simulink model "CCMSysServ"  (R2022b)
settings: Solver=FixedStepDiscrete  StartTime=0.0  StopTime=10.0  FixedStep=0.05  RelTol=1e-3
model workspace (MAT data in the model file):
  NvMBlockCarCfgData : Simulink.Signal (value not decoded)
  NvMBlockCarCfgDataInit : Simulink.Parameter (value not decoded)
  NvMBlockDidDataD005 : Simulink.Signal (value not decoded)
  NvMBlockDidDataD005Init : Simulink.Parameter (value not decoded)
  NvMBlockDidDataD09A : Simulink.Signal (value not decoded)
  NvMBlockDidDataD09AInit : Simulink.Parameter (value not decoded)
  NvMBlockDidDataE103 : Simulink.Signal (value not decoded)
  NvMBlockDidDataE103Init : Simulink.Parameter (value not decoded)
  NvMBlockDidDataF190 : Simulink.Signal (value not decoded)
  NvMBlockDidDataF190Init : Simulink.Parameter (value not decoded)
  NvMBlockDidDataF1AB : Simulink.Signal (value not decoded)
  NvMBlockDidDataF1ABInit : Simulink.Parameter (value not decoded)
symbols that are objects in the model workspace (value not decoded): NvMBlockCarCfgDataInit, NvMBlockDidDataD005Init, NvMBlockDidDataD09AInit, NvMBlockDidDataE103Init, NvMBlockDidDataF190Init, NvMBlockDidDataF1ABInit
(part 3 of 4)

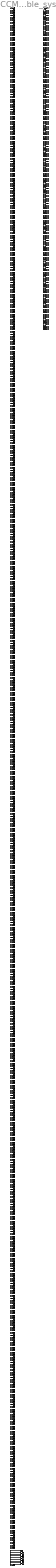
== system CCMSysServ/CCMSysServ_Runnable_sys ==
  ComMPNCState_PNCState_read : Inport
  ComMPNCState_VFCState_read : Inport  Port=2
  EcuKeepActv_EcuKeepActv_read : Inport  Port=3
  CarTiGlb_CarTiGlb_read : Inport  Port=4
  CarTiGlb_CarTiGlb_IsUpdated_read : Inport  Port=5
  ActrFrntReqForRowFirstLeModDistbn_ActrFrntReqForRowFirstLeModDistbn_read : Inport  Port=6
  CarCfg_CarCfg1_read : Inport  Port=7
  CarCfg_CarCfg10_read : Inport  Port=8
  CarCfg_CarCfg100_read : Inport  Port=9
  CarCfg_CarCfg1002_read : Inport  Port=10
  CarCfg_CarCfg1004_read : Inport  Port=11
  CarCfg_CarCfg1007_read : Inport  Port=12
  CarCfg_CarCfg101_read : Inport  Port=13
  CarCfg_CarCfg1011_read : Inport  Port=14
  CarCfg_CarCfg1017_read : Inport  Port=15
  CarCfg_CarCfg1018_read : Inport  Port=16
  CarCfg_CarCfg102_read : Inport  Port=17
  CarCfg_CarCfg103_read : Inport  Port=18
  CarCfg_CarCfg1034_read : Inport  Port=19
  CarCfg_CarCfg1038_read : Inport  Port=20
  CarCfg_CarCfg1039_read : Inport  Port=21
  CarCfg_CarCfg104_read : Inport  Port=22
  CarCfg_CarCfg1042_read : Inport  Port=23
  CarCfg_CarCfg1043_read : Inport  Port=24
  CarCfg_CarCfg1044_read : Inport  Port=25
  CarCfg_CarCfg1045_read : Inport  Port=26
  CarCfg_CarCfg1047_read : Inport  Port=27
  CarCfg_CarCfg105_read : Inport  Port=28
  CarCfg_CarCfg1052_read : Inport  Port=29
  CarCfg_CarCfg1057_read : Inport  Port=30
  CarCfg_CarCfg106_read : Inport  Port=31
  CarCfg_CarCfg1061_read : Inport  Port=32
  CarCfg_CarCfg1063_read : Inport  Port=33
  CarCfg_CarCfg1065_read : Inport  Port=34
  CarCfg_CarCfg107_read : Inport  Port=35
  CarCfg_CarCfg1075_read : Inport  Port=36
  CarCfg_CarCfg1077_read : Inport  Port=37
  CarCfg_CarCfg108_read : Inport  Port=38
  CarCfg_CarCfg1080_read : Inport  Port=39
  CarCfg_CarCfg1083_read : Inport  Port=40
  CarCfg_CarCfg1087_read : Inport  Port=41
  CarCfg_CarCfg1088_read : Inport  Port=42
  CarCfg_CarCfg109_read : Inport  Port=43
  CarCfg_CarCfg1090_read : Inport  Port=44
  CarCfg_CarCfg1096_read : Inport  Port=45
  CarCfg_CarCfg110_read : Inport  Port=46
  CarCfg_CarCfg1101_read : Inport  Port=47
  CarCfg_CarCfg1102_read : Inport  Port=48
  CarCfg_CarCfg1103_read : Inport  Port=49
  CarCfg_CarCfg1104_read : Inport  Port=50
  CarCfg_CarCfg111_read : Inport  Port=51
  CarCfg_CarCfg1111_read : Inport  Port=52
  CarCfg_CarCfg1112_read : Inport  Port=53
  CarCfg_CarCfg112_read : Inport  Port=54
  CarCfg_CarCfg113_read : Inport  Port=55
  CarCfg_CarCfg1130_read : Inport  Port=56
  CarCfg_CarCfg1134_read : Inport  Port=57
  CarCfg_CarCfg1136_read : Inport  Port=58
  CarCfg_CarCfg114_read : Inport  Port=59
  CarCfg_CarCfg115_read : Inport  Port=60
  CarCfg_CarCfg1154_read : Inport  Port=61
  CarCfg_CarCfg116_read : Inport  Port=62
  CarCfg_CarCfg1166_read : Inport  Port=63
  CarCfg_CarCfg117_read : Inport  Port=64
  CarCfg_CarCfg1170_read : Inport  Port=65
  CarCfg_CarCfg1171_read : Inport  Port=66
  CarCfg_CarCfg1172_read : Inport  Port=67
  CarCfg_CarCfg1173_read : Inport  Port=68
  CarCfg_CarCfg1174_read : Inport  Port=69
  CarCfg_CarCfg1175_read : Inport  Port=70
  CarCfg_CarCfg1177_read : Inport  Port=71
  CarCfg_CarCfg1178_read : Inport  Port=72
  CarCfg_CarCfg118_read : Inport  Port=73
  CarCfg_CarCfg1184_read : Inport  Port=74
  CarCfg_CarCfg1185_read : Inport  Port=75
  CarCfg_CarCfg1189_read : Inport  Port=76
  CarCfg_CarCfg119_read : Inport  Port=77
  CarCfg_CarCfg12_read : Inport  Port=78
  CarCfg_CarCfg120_read : Inport  Port=79
  CarCfg_CarCfg121_read : Inport  Port=80
  CarCfg_CarCfg1214_read : Inport  Port=81
  CarCfg_CarCfg1216_read : Inport  Port=82
  CarCfg_CarCfg1217_read : Inport  Port=83
  CarCfg_CarCfg1218_read : Inport  Port=84
  CarCfg_CarCfg1219_read : Inport  Port=85
  CarCfg_CarCfg122_read : Inport  Port=86
  CarCfg_CarCfg123_read : Inport  Port=87
  CarCfg_CarCfg1230_read : Inport  Port=88
  CarCfg_CarCfg1231_read : Inport  Port=89
  CarCfg_CarCfg1232_read : Inport  Port=90
  CarCfg_CarCfg1233_read : Inport  Port=91
  CarCfg_CarCfg1234_read : Inport  Port=92
  CarCfg_CarCfg124_read : Inport  Port=93
  CarCfg_CarCfg1247_read : Inport  Port=94
  CarCfg_CarCfg125_read : Inport  Port=95
  CarCfg_CarCfg1250_read : Inport  Port=96
  CarCfg_CarCfg1251_read : Inport  Port=97
  CarCfg_CarCfg1258_read : Inport  Port=98
  CarCfg_CarCfg1259_read : Inport  Port=99
  CarCfg_CarCfg126_read : Inport  Port=100
  CarCfg_CarCfg1260_read : Inport  Port=101
  CarCfg_CarCfg1261_read : Inport  Port=102
  CarCfg_CarCfg1264_read : Inport  Port=103
  CarCfg_CarCfg1266_read : Inport  Port=104
  CarCfg_CarCfg1267_read : Inport  Port=105
  CarCfg_CarCfg127_read : Inport  Port=106
  CarCfg_CarCfg1273_read : Inport  Port=107
  CarCfg_CarCfg1275_read : Inport  Port=108
  CarCfg_CarCfg1277_read : Inport  Port=109
  CarCfg_CarCfg1278_read : Inport  Port=110
  CarCfg_CarCfg1279_read : Inport  Port=111
  CarCfg_CarCfg128_read : Inport  Port=112
  CarCfg_CarCfg1283_read : Inport  Port=113
  CarCfg_CarCfg1285_read : Inport  Port=114
  CarCfg_CarCfg1286_read : Inport  Port=115
  CarCfg_CarCfg1287_read : Inport  Port=116
  CarCfg_CarCfg129_read : Inport  Port=117
  CarCfg_CarCfg13_read : Inport  Port=118
  CarCfg_CarCfg130_read : Inport  Port=119
  CarCfg_CarCfg131_read : Inport  Port=120
  CarCfg_CarCfg132_read : Inport  Port=121
  CarCfg_CarCfg133_read : Inport  Port=122
  CarCfg_CarCfg134_read : Inport  Port=123
  CarCfg_CarCfg135_read : Inport  Port=124
  CarCfg_CarCfg136_read : Inport  Port=125
  CarCfg_CarCfg137_read : Inport  Port=126
  CarCfg_CarCfg138_read : Inport  Port=127
  CarCfg_CarCfg139_read : Inport  Port=128
  CarCfg_CarCfg14_read : Inport  Port=129
  CarCfg_CarCfg140_read : Inport  Port=130
  CarCfg_CarCfg141_read : Inport  Port=131
  CarCfg_CarCfg142_read : Inport  Port=132
  CarCfg_CarCfg143_read : Inport  Port=133
  CarCfg_CarCfg144_read : Inport  Port=134
  CarCfg_CarCfg145_read : Inport  Port=135
  CarCfg_CarCfg146_read : Inport  Port=136
  CarCfg_CarCfg148_read : Inport  Port=137
  CarCfg_CarCfg149_read : Inport  Port=138
  CarCfg_CarCfg150_read : Inport  Port=139
  CarCfg_CarCfg151_read : Inport  Port=140
  CarCfg_CarCfg152_read : Inport  Port=141
  CarCfg_CarCfg153_read : Inport  Port=142
  CarCfg_CarCfg154_read : Inport  Port=143
  CarCfg_CarCfg155_read : Inport  Port=144
  CarCfg_CarCfg156_read : Inport  Port=145
  CarCfg_CarCfg157_read : Inport  Port=146
  CarCfg_CarCfg158_read : Inport  Port=147
  CarCfg_CarCfg159_read : Inport  Port=148
  CarCfg_CarCfg16_read : Inport  Port=149
  CarCfg_CarCfg160_read : Inport  Port=150
  CarCfg_CarCfg161_read : Inport  Port=151
  CarCfg_CarCfg162_read : Inport  Port=152
  CarCfg_CarCfg163_read : Inport  Port=153
  CarCfg_CarCfg164_read : Inport  Port=154
  CarCfg_CarCfg165_read : Inport  Port=155
  CarCfg_CarCfg166_read : Inport  Port=156
  CarCfg_CarCfg167_read : Inport  Port=157
  CarCfg_CarCfg168_read : Inport  Port=158
  CarCfg_CarCfg169_read : Inport  Port=159
  CarCfg_CarCfg17_read : Inport  Port=160
  CarCfg_CarCfg170_read : Inport  Port=161
  CarCfg_CarCfg171_read : Inport  Port=162
  CarCfg_CarCfg172_read : Inport  Port=163
  CarCfg_CarCfg173_read : Inport  Port=164
  CarCfg_CarCfg174_read : Inport  Port=165
  CarCfg_CarCfg175_read : Inport  Port=166
  CarCfg_CarCfg176_read : Inport  Port=167
  CarCfg_CarCfg177_read : Inport  Port=168
  CarCfg_CarCfg178_read : Inport  Port=169
  CarCfg_CarCfg179_read : Inport  Port=170
  CarCfg_CarCfg18_read : Inport  Port=171
  CarCfg_CarCfg180_read : Inport  Port=172
  CarCfg_CarCfg181_read : Inport  Port=173
  CarCfg_CarCfg182_read : Inport  Port=174
  CarCfg_CarCfg183_read : Inport  Port=175
  CarCfg_CarCfg184_read : Inport  Port=176
  CarCfg_CarCfg185_read : Inport  Port=177
  CarCfg_CarCfg186_read : Inport  Port=178
  CarCfg_CarCfg187_read : Inport  Port=179
  CarCfg_CarCfg188_read : Inport  Port=180
  CarCfg_CarCfg189_read : Inport  Port=181
  CarCfg_CarCfg19_read : Inport  Port=182
  CarCfg_CarCfg190_read : Inport  Port=183
  CarCfg_CarCfg191_read : Inport  Port=184
  CarCfg_CarCfg192_read : Inport  Port=185
  CarCfg_CarCfg193_read : Inport  Port=186
  CarCfg_CarCfg194_read : Inport  Port=187
  CarCfg_CarCfg195_read : Inport  Port=188
  CarCfg_CarCfg196_read : Inport  Port=189
  CarCfg_CarCfg197_read : Inport  Port=190
  CarCfg_CarCfg198_read : Inport  Port=191
  CarCfg_CarCfg199_read : Inport  Port=192
  CarCfg_CarCfg2_read : Inport  Port=193
  CarCfg_CarCfg20_read : Inport  Port=194
  CarCfg_CarCfg200_read : Inport  Port=195
  CarCfg_CarCfg201_read : Inport  Port=196
  CarCfg_CarCfg202_read : Inport  Port=197
  CarCfg_CarCfg203_read : Inport  Port=198
  CarCfg_CarCfg204_read : Inport  Port=199
  CarCfg_CarCfg205_read : Inport  Port=200
  CarCfg_CarCfg206_read : Inport  Port=201
  CarCfg_CarCfg207_read : Inport  Port=202
  CarCfg_CarCfg208_read : Inport  Port=203
  CarCfg_CarCfg209_read : Inport  Port=204
  CarCfg_CarCfg210_read : Inport  Port=205
  CarCfg_CarCfg211_read : Inport  Port=206
  CarCfg_CarCfg212_read : Inport  Port=207
  CarCfg_CarCfg213_read : Inport  Port=208
  CarCfg_CarCfg214_read : Inport  Port=209
  CarCfg_CarCfg215_read : Inport  Port=210
  CarCfg_CarCfg216_read : Inport  Port=211
  CarCfg_CarCfg217_read : Inport  Port=212
  CarCfg_CarCfg218_read : Inport  Port=213
  CarCfg_CarCfg219_read : Inport  Port=214
  CarCfg_CarCfg22_read : Inport  Port=215
  CarCfg_CarCfg220_read : Inport  Port=216
  CarCfg_CarCfg221_read : Inport  Port=217
  CarCfg_CarCfg222_read : Inport  Port=218
  CarCfg_CarCfg223_read : Inport  Port=219
  CarCfg_CarCfg224_read : Inport  Port=220
  CarCfg_CarCfg225_read : Inport  Port=221
  CarCfg_CarCfg226_read : Inport  Port=222
  CarCfg_CarCfg227_read : Inport  Port=223
  CarCfg_CarCfg228_read : Inport  Port=224
  CarCfg_CarCfg229_read : Inport  Port=225
  CarCfg_CarCfg23_read : Inport  Port=226
  CarCfg_CarCfg230_read : Inport  Port=227
  CarCfg_CarCfg231_read : Inport  Port=228
  CarCfg_CarCfg232_read : Inport  Port=229
  CarCfg_CarCfg235_read : Inport  Port=230
  CarCfg_CarCfg236_read : Inport  Port=231
  CarCfg_CarCfg237_read : Inport  Port=232
  CarCfg_CarCfg238_read : Inport  Port=233
  CarCfg_CarCfg239_read : Inport  Port=234
  CarCfg_CarCfg24_read : Inport  Port=235
  CarCfg_CarCfg240_read : Inport  Port=236
  CarCfg_CarCfg241_read : Inport  Port=237
  CarCfg_CarCfg242_read : Inport  Port=238
  CarCfg_CarCfg243_read : Inport  Port=239
  CarCfg_CarCfg244_read : Inport  Port=240
  CarCfg_CarCfg245_read : Inport  Port=241
  CarCfg_CarCfg246_read : Inport  Port=242
  CarCfg_CarCfg247_read : Inport  Port=243
  CarCfg_CarCfg248_read : Inport  Port=244
  CarCfg_CarCfg249_read : Inport  Port=245
  CarCfg_CarCfg25_read : Inport  Port=246
  CarCfg_CarCfg250_read : Inport  Port=247
  CarCfg_CarCfg253_read : Inport  Port=248
  CarCfg_CarCfg254_read : Inport  Port=249
  CarCfg_CarCfg255_read : Inport  Port=250
  CarCfg_CarCfg256_read : Inport  Port=251
  CarCfg_CarCfg257_read : Inport  Port=252
  CarCfg_CarCfg258_read : Inport  Port=253
  CarCfg_CarCfg259_read : Inport  Port=254
  CarCfg_CarCfg262_read : Inport  Port=255
  CarCfg_CarCfg263_read : Inport  Port=256
  CarCfg_CarCfg267_read : Inport  Port=257
  CarCfg_CarCfg268_read : Inport  Port=258
  CarCfg_CarCfg270_read : Inport  Port=259
  CarCfg_CarCfg271_read : Inport  Port=260
  CarCfg_CarCfg273_read : Inport  Port=261
  CarCfg_CarCfg274_read : Inport  Port=262
  CarCfg_CarCfg275_read : Inport  Port=263
  CarCfg_CarCfg28_read : Inport  Port=264
  CarCfg_CarCfg289_read : Inport  Port=265
  CarCfg_CarCfg290_read : Inport  Port=266
  CarCfg_CarCfg3_read : Inport  Port=267
  CarCfg_CarCfg30_read : Inport  Port=268
  CarCfg_CarCfg301_read : Inport  Port=269
  CarCfg_CarCfg302_read : Inport  Port=270
  CarCfg_CarCfg303_read : Inport  Port=271
  CarCfg_CarCfg304_read : Inport  Port=272
  CarCfg_CarCfg305_read : Inport  Port=273
  CarCfg_CarCfg307_read : Inport  Port=274
  CarCfg_CarCfg308_read : Inport  Port=275
  CarCfg_CarCfg309_read : Inport  Port=276
  CarCfg_CarCfg310_read : Inport  Port=277
  CarCfg_CarCfg311_read : Inport  Port=278
  CarCfg_CarCfg312_read : Inport  Port=279
  CarCfg_CarCfg313_read : Inport  Port=280
  CarCfg_CarCfg314_read : Inport  Port=281
  CarCfg_CarCfg315_read : Inport  Port=282
  CarCfg_CarCfg316_read : Inport  Port=283
  CarCfg_CarCfg317_read : Inport  Port=284
  CarCfg_CarCfg318_read : Inport  Port=285
  CarCfg_CarCfg319_read : Inport  Port=286
  CarCfg_CarCfg32_read : Inport  Port=287
  CarCfg_CarCfg321_read : Inport  Port=288
  CarCfg_CarCfg322_read : Inport  Port=289
  CarCfg_CarCfg323_read : Inport  Port=290
  CarCfg_CarCfg324_read : Inport  Port=291
  CarCfg_CarCfg325_read : Inport  Port=292
  CarCfg_CarCfg33_read : Inport  Port=293
  CarCfg_CarCfg335_read : Inport  Port=294
  CarCfg_CarCfg336_read : Inport  Port=295
  CarCfg_CarCfg337_read : Inport  Port=296
  CarCfg_CarCfg338_read : Inport  Port=297
  CarCfg_CarCfg339_read : Inport  Port=298
  CarCfg_CarCfg34_read : Inport  Port=299
  CarCfg_CarCfg340_read : Inport  Port=300
  CarCfg_CarCfg341_read : Inport  Port=301
  CarCfg_CarCfg342_read : Inport  Port=302
  CarCfg_CarCfg343_read : Inport  Port=303
  CarCfg_CarCfg344_read : Inport  Port=304
  CarCfg_CarCfg345_read : Inport  Port=305
  CarCfg_CarCfg346_read : Inport  Port=306
  CarCfg_CarCfg347_read : Inport  Port=307
  CarCfg_CarCfg348_read : Inport  Port=308
  CarCfg_CarCfg349_read : Inport  Port=309
  CarCfg_CarCfg35_read : Inport  Port=310
  CarCfg_CarCfg350_read : Inport  Port=311
  CarCfg_CarCfg351_read : Inport  Port=312
  CarCfg_CarCfg352_read : Inport  Port=313
  CarCfg_CarCfg353_read : Inport  Port=314
  CarCfg_CarCfg354_read : Inport  Port=315
  CarCfg_CarCfg355_read : Inport  Port=316
  CarCfg_CarCfg356_read : Inport  Port=317
  CarCfg_CarCfg357_read : Inport  Port=318
  CarCfg_CarCfg358_read : Inport  Port=319
  CarCfg_CarCfg359_read : Inport  Port=320
  CarCfg_CarCfg36_read : Inport  Port=321
  CarCfg_CarCfg360_read : Inport  Port=322
  CarCfg_CarCfg361_read : Inport  Port=323
  CarCfg_CarCfg362_read : Inport  Port=324
  CarCfg_CarCfg363_read : Inport  Port=325
  CarCfg_CarCfg364_read : Inport  Port=326
  CarCfg_CarCfg365_read : Inport  Port=327
  CarCfg_CarCfg366_read : Inport  Port=328
  CarCfg_CarCfg367_read : Inport  Port=329
  CarCfg_CarCfg368_read : Inport  Port=330
  CarCfg_CarCfg369_read : Inport  Port=331
  CarCfg_CarCfg37_read : Inport  Port=332
  CarCfg_CarCfg371_read : Inport  Port=333
  CarCfg_CarCfg372_read : Inport  Port=334
  CarCfg_CarCfg373_read : Inport  Port=335
  CarCfg_CarCfg374_read : Inport  Port=336
  CarCfg_CarCfg376_read : Inport  Port=337
  CarCfg_CarCfg38_read : Inport  Port=338
  CarCfg_CarCfg39_read : Inport  Port=339
  CarCfg_CarCfg4_read : Inport  Port=340
  CarCfg_CarCfg401_read : Inport  Port=341
  CarCfg_CarCfg407_read : Inport  Port=342
  CarCfg_CarCfg408_read : Inport  Port=343
  CarCfg_CarCfg409_read : Inport  Port=344
  CarCfg_CarCfg41_read : Inport  Port=345
  CarCfg_CarCfg410_read : Inport  Port=346
  CarCfg_CarCfg413_read : Inport  Port=347
  CarCfg_CarCfg414_read : Inport  Port=348
  CarCfg_CarCfg415_read : Inport  Port=349
  CarCfg_CarCfg42_read : Inport  Port=350
  CarCfg_CarCfg43_read : Inport  Port=351
  CarCfg_CarCfg44_read : Inport  Port=352
  CarCfg_CarCfg441_read : Inport  Port=353
  CarCfg_CarCfg449_read : Inport  Port=354
  CarCfg_CarCfg45_read : Inport  Port=355
  CarCfg_CarCfg450_read : Inport  Port=356
  CarCfg_CarCfg451_read : Inport  Port=357
  CarCfg_CarCfg452_read : Inport  Port=358
  CarCfg_CarCfg453_read : Inport  Port=359
  CarCfg_CarCfg454_read : Inport  Port=360
  CarCfg_CarCfg455_read : Inport  Port=361
  CarCfg_CarCfg456_read : Inport  Port=362
  CarCfg_CarCfg457_read : Inport  Port=363
  CarCfg_CarCfg458_read : Inport  Port=364
  CarCfg_CarCfg459_read : Inport  Port=365
  CarCfg_CarCfg46_read : Inport  Port=366
  CarCfg_CarCfg460_read : Inport  Port=367
  CarCfg_CarCfg461_read : Inport  Port=368
  CarCfg_CarCfg462_read : Inport  Port=369
  CarCfg_CarCfg463_read : Inport  Port=370
  CarCfg_CarCfg464_read : Inport  Port=371
  CarCfg_CarCfg465_read : Inport  Port=372
  CarCfg_CarCfg466_read : Inport  Port=373
  CarCfg_CarCfg467_read : Inport  Port=374
  CarCfg_CarCfg468_read : Inport  Port=375
  CarCfg_CarCfg469_read : Inport  Port=376
  CarCfg_CarCfg47_read : Inport  Port=377
  CarCfg_CarCfg470_read : Inport  Port=378
  CarCfg_CarCfg471_read : Inport  Port=379
  CarCfg_CarCfg472_read : Inport  Port=380
  CarCfg_CarCfg473_read : Inport  Port=381
  CarCfg_CarCfg474_read : Inport  Port=382
  CarCfg_CarCfg475_read : Inport  Port=383
  CarCfg_CarCfg476_read : Inport  Port=384
  CarCfg_CarCfg477_read : Inport  Port=385
  CarCfg_CarCfg478_read : Inport  Port=386
  CarCfg_CarCfg479_read : Inport  Port=387
  CarCfg_CarCfg48_read : Inport  Port=388
  CarCfg_CarCfg480_read : Inport  Port=389
  CarCfg_CarCfg481_read : Inport  Port=390
  CarCfg_CarCfg482_read : Inport  Port=391
  CarCfg_CarCfg483_read : Inport  Port=392
  CarCfg_CarCfg484_read : Inport  Port=393
  CarCfg_CarCfg485_read : Inport  Port=394
  CarCfg_CarCfg486_read : Inport  Port=395
  CarCfg_CarCfg487_read : Inport  Port=396
  CarCfg_CarCfg488_read : Inport  Port=397
  CarCfg_CarCfg489_read : Inport  Port=398
  CarCfg_CarCfg49_read : Inport  Port=399
  CarCfg_CarCfg490_read : Inport  Port=400
  CarCfg_CarCfg491_read : Inport  Port=401
  CarCfg_CarCfg493_read : Inport  Port=402
  CarCfg_CarCfg494_read : Inport  Port=403
  CarCfg_CarCfg495_read : Inport  Port=404
  CarCfg_CarCfg496_read : Inport  Port=405
  CarCfg_CarCfg497_read : Inport  Port=406
  CarCfg_CarCfg498_read : Inport  Port=407
  CarCfg_CarCfg499_read : Inport  Port=408
  CarCfg_CarCfg5_read : Inport  Port=409
  CarCfg_CarCfg50_read : Inport  Port=410
  CarCfg_CarCfg500_read : Inport  Port=411
  CarCfg_CarCfg501_read : Inport  Port=412
  CarCfg_CarCfg502_read : Inport  Port=413
  CarCfg_CarCfg503_read : Inport  Port=414
  CarCfg_CarCfg504_read : Inport  Port=415
  CarCfg_CarCfg505_read : Inport  Port=416
  CarCfg_CarCfg506_read : Inport  Port=417
  CarCfg_CarCfg507_read : Inport  Port=418
  CarCfg_CarCfg508_read : Inport  Port=419
  CarCfg_CarCfg509_read : Inport  Port=420
  CarCfg_CarCfg51_read : Inport  Port=421
  CarCfg_CarCfg510_read : Inport  Port=422
  CarCfg_CarCfg511_read : Inport  Port=423
  CarCfg_CarCfg512_read : Inport  Port=424
  CarCfg_CarCfg513_read : Inport  Port=425
  CarCfg_CarCfg514_read : Inport  Port=426
  CarCfg_CarCfg515_read : Inport  Port=427
  CarCfg_CarCfg516_read : Inport  Port=428
  CarCfg_CarCfg517_read : Inport  Port=429
  CarCfg_CarCfg518_read : Inport  Port=430
  CarCfg_CarCfg519_read : Inport  Port=431
  CarCfg_CarCfg52_read : Inport  Port=432
  CarCfg_CarCfg520_read : Inport  Port=433
  CarCfg_CarCfg521_read : Inport  Port=434
  CarCfg_CarCfg522_read : Inport  Port=435
  CarCfg_CarCfg523_read : Inport  Port=436
  CarCfg_CarCfg524_read : Inport  Port=437
  CarCfg_CarCfg525_read : Inport  Port=438
  CarCfg_CarCfg526_read : Inport  Port=439
  CarCfg_CarCfg527_read : Inport  Port=440
  CarCfg_CarCfg528_read : Inport  Port=441
  CarCfg_CarCfg529_read : Inport  Port=442
  CarCfg_CarCfg53_read : Inport  Port=443
  CarCfg_CarCfg531_read : Inport  Port=444
  CarCfg_CarCfg532_read : Inport  Port=445
  CarCfg_CarCfg533_read : Inport  Port=446
  CarCfg_CarCfg534_read : Inport  Port=447
  CarCfg_CarCfg535_read : Inport  Port=448
  CarCfg_CarCfg536_read : Inport  Port=449
  CarCfg_CarCfg54_read : Inport  Port=450
  CarCfg_CarCfg540_read : Inport  Port=451
  CarCfg_CarCfg541_read : Inport  Port=452
  CarCfg_CarCfg543_read : Inport  Port=453
  CarCfg_CarCfg544_read : Inport  Port=454
  CarCfg_CarCfg545_read : Inport  Port=455
  CarCfg_CarCfg546_read : Inport  Port=456
  CarCfg_CarCfg547_read : Inport  Port=457
  CarCfg_CarCfg55_read : Inport  Port=458
  CarCfg_CarCfg550_read : Inport  Port=459
  CarCfg_CarCfg551_read : Inport  Port=460
  CarCfg_CarCfg56_read : Inport  Port=461
  CarCfg_CarCfg561_read : Inport  Port=462
  CarCfg_CarCfg564_read : Inport  Port=463
  CarCfg_CarCfg565_read : Inport  Port=464
  CarCfg_CarCfg566_read : Inport  Port=465
  CarCfg_CarCfg567_read : Inport  Port=466
  CarCfg_CarCfg568_read : Inport  Port=467
  CarCfg_CarCfg569_read : Inport  Port=468
  CarCfg_CarCfg57_read : Inport  Port=469
  CarCfg_CarCfg578_read : Inport  Port=470
  CarCfg_CarCfg579_read : Inport  Port=471
  CarCfg_CarCfg58_read : Inport  Port=472
  CarCfg_CarCfg580_read : Inport  Port=473
  CarCfg_CarCfg59_read : Inport  Port=474
  CarCfg_CarCfg590_read : Inport  Port=475
  CarCfg_CarCfg591_read : Inport  Port=476
  CarCfg_CarCfg593_read : Inport  Port=477
  CarCfg_CarCfg595_read : Inport  Port=478
  CarCfg_CarCfg599_read : Inport  Port=479
  CarCfg_CarCfg6_read : Inport  Port=480
  CarCfg_CarCfg60_read : Inport  Port=481
  CarCfg_CarCfg604_read : Inport  Port=482
  CarCfg_CarCfg609_read : Inport  Port=483
  CarCfg_CarCfg61_read : Inport  Port=484
  CarCfg_CarCfg611_read : Inport  Port=485
  CarCfg_CarCfg613_read : Inport  Port=486
  CarCfg_CarCfg615_read : Inport  Port=487
  CarCfg_CarCfg616_read : Inport  Port=488
  CarCfg_CarCfg617_read : Inport  Port=489
  CarCfg_CarCfg618_read : Inport  Port=490
  CarCfg_CarCfg62_read : Inport  Port=491
  CarCfg_CarCfg622_read : Inport  Port=492
  CarCfg_CarCfg624_read : Inport  Port=493
  CarCfg_CarCfg626_read : Inport  Port=494
  CarCfg_CarCfg627_read : Inport  Port=495
  CarCfg_CarCfg628_read : Inport  Port=496
  CarCfg_CarCfg629_read : Inport  Port=497
  CarCfg_CarCfg63_read : Inport  Port=498
  CarCfg_CarCfg630_read : Inport  Port=499
  CarCfg_CarCfg634_read : Inport  Port=500
  CarCfg_CarCfg635_read : Inport  Port=501
  CarCfg_CarCfg639_read : Inport  Port=502
  CarCfg_CarCfg64_read : Inport  Port=503
  CarCfg_CarCfg640_read : Inport  Port=504
  CarCfg_CarCfg642_read : Inport  Port=505
  CarCfg_CarCfg644_read : Inport  Port=506
  CarCfg_CarCfg645_read : Inport  Port=507
  CarCfg_CarCfg646_read : Inport  Port=508
  CarCfg_CarCfg647_read : Inport  Port=509
  CarCfg_CarCfg648_read : Inport  Port=510
  CarCfg_CarCfg65_read : Inport  Port=511
  CarCfg_CarCfg650_read : Inport  Port=512
  CarCfg_CarCfg651_read : Inport  Port=513
  CarCfg_CarCfg652_read : Inport  Port=514
  CarCfg_CarCfg659_read : Inport  Port=515
  CarCfg_CarCfg66_read : Inport  Port=516
  CarCfg_CarCfg660_read : Inport  Port=517
  CarCfg_CarCfg665_read : Inport  Port=518
  CarCfg_CarCfg67_read : Inport  Port=519
  CarCfg_CarCfg671_read : Inport  Port=520
  CarCfg_CarCfg672_read : Inport  Port=521
  CarCfg_CarCfg675_read : Inport  Port=522
  CarCfg_CarCfg677_read : Inport  Port=523
  CarCfg_CarCfg678_read : Inport  Port=524
  CarCfg_CarCfg68_read : Inport  Port=525
  CarCfg_CarCfg683_read : Inport  Port=526
  CarCfg_CarCfg684_read : Inport  Port=527
  CarCfg_CarCfg686_read : Inport  Port=528
  CarCfg_CarCfg687_read : Inport  Port=529
  CarCfg_CarCfg688_read : Inport  Port=530
  CarCfg_CarCfg69_read : Inport  Port=531
  CarCfg_CarCfg695_read : Inport  Port=532
  CarCfg_CarCfg697_read : Inport  Port=533
  CarCfg_CarCfg698_read : Inport  Port=534
  CarCfg_CarCfg699_read : Inport  Port=535
  CarCfg_CarCfg70_read : Inport  Port=536
  CarCfg_CarCfg701_read : Inport  Port=537
  CarCfg_CarCfg705_read : Inport  Port=538
  CarCfg_CarCfg709_read : Inport  Port=539
  CarCfg_CarCfg71_read : Inport  Port=540
  CarCfg_CarCfg714_read : Inport  Port=541
  CarCfg_CarCfg715_read : Inport  Port=542
  CarCfg_CarCfg718_read : Inport  Port=543
  CarCfg_CarCfg719_read : Inport  Port=544
  CarCfg_CarCfg72_read : Inport  Port=545
  CarCfg_CarCfg720_read : Inport  Port=546
  CarCfg_CarCfg721_read : Inport  Port=547
  CarCfg_CarCfg726_read : Inport  Port=548
  CarCfg_CarCfg73_read : Inport  Port=549
  CarCfg_CarCfg732_read : Inport  Port=550
  CarCfg_CarCfg733_read : Inport  Port=551
  CarCfg_CarCfg734_read : Inport  Port=552
  CarCfg_CarCfg74_read : Inport  Port=553
  CarCfg_CarCfg740_read : Inport  Port=554
  CarCfg_CarCfg741_read : Inport  Port=555
  CarCfg_CarCfg742_read : Inport  Port=556
  CarCfg_CarCfg746_read : Inport  Port=557
  CarCfg_CarCfg75_read : Inport  Port=558
  CarCfg_CarCfg753_read : Inport  Port=559
  CarCfg_CarCfg755_read : Inport  Port=560
  CarCfg_CarCfg756_read : Inport  Port=561
  CarCfg_CarCfg76_read : Inport  Port=562
  CarCfg_CarCfg763_read : Inport  Port=563
  CarCfg_CarCfg766_read : Inport  Port=564
  CarCfg_CarCfg768_read : Inport  Port=565
  CarCfg_CarCfg77_read : Inport  Port=566
  CarCfg_CarCfg770_read : Inport  Port=567
  CarCfg_CarCfg773_read : Inport  Port=568
  CarCfg_CarCfg776_read : Inport  Port=569
  CarCfg_CarCfg779_read : Inport  Port=570
  CarCfg_CarCfg78_read : Inport  Port=571
  CarCfg_CarCfg787_read : Inport  Port=572
  CarCfg_CarCfg789_read : Inport  Port=573
  CarCfg_CarCfg79_read : Inport  Port=574
  CarCfg_CarCfg790_read : Inport  Port=575
  CarCfg_CarCfg793_read : Inport  Port=576
  CarCfg_CarCfg794_read : Inport  Port=577
  CarCfg_CarCfg798_read : Inport  Port=578
  CarCfg_CarCfg8_read : Inport  Port=579
  CarCfg_CarCfg80_read : Inport  Port=580
  CarCfg_CarCfg81_read : Inport  Port=581
  CarCfg_CarCfg811_read : Inport  Port=582
  CarCfg_CarCfg814_read : Inport  Port=583
  CarCfg_CarCfg816_read : Inport  Port=584
  CarCfg_CarCfg82_read : Inport  Port=585
  CarCfg_CarCfg822_read : Inport  Port=586
  CarCfg_CarCfg826_read : Inport  Port=587
  CarCfg_CarCfg829_read : Inport  Port=588
  CarCfg_CarCfg83_read : Inport  Port=589
  CarCfg_CarCfg831_read : Inport  Port=590
  CarCfg_CarCfg834_read : Inport  Port=591
  CarCfg_CarCfg835_read : Inport  Port=592
  CarCfg_CarCfg837_read : Inport  Port=593
  CarCfg_CarCfg839_read : Inport  Port=594
  CarCfg_CarCfg84_read : Inport  Port=595
  CarCfg_CarCfg841_read : Inport  Port=596
  CarCfg_CarCfg842_read : Inport  Port=597
  CarCfg_CarCfg846_read : Inport  Port=598
  CarCfg_CarCfg848_read : Inport  Port=599
  CarCfg_CarCfg849_read : Inport  Port=600
  CarCfg_CarCfg85_read : Inport  Port=601
  CarCfg_CarCfg851_read : Inport  Port=602
  CarCfg_CarCfg855_read : Inport  Port=603
  CarCfg_CarCfg857_read : Inport  Port=604
  CarCfg_CarCfg858_read : Inport  Port=605
  CarCfg_CarCfg86_read : Inport  Port=606
  CarCfg_CarCfg87_read : Inport  Port=607
  CarCfg_CarCfg88_read : Inport  Port=608
  CarCfg_CarCfg89_read : Inport  Port=609
  CarCfg_CarCfg9_read : Inport  Port=610
  CarCfg_CarCfg90_read : Inport  Port=611
  CarCfg_CarCfg91_read : Inport  Port=612
  CarCfg_CarCfg92_read : Inport  Port=613
  CarCfg_CarCfg93_read : Inport  Port=614
  CarCfg_CarCfg94_read : Inport  Port=615
  CarCfg_CarCfg95_read : Inport  Port=616
  CarCfg_CarCfg96_read : Inport  Port=617
  CarCfg_CarCfg97_read : Inport  Port=618
  CarCfg_CarCfg98_read : Inport  Port=619
  CarCfg_CarCfg99_read : Inport  Port=620
  VehCfgPrm_VehCfgPrm_read : Inport  Port=621
  VehCfgPrmExt_VehCfgPrmExt_read : Inport  Port=622
  VehModMngtGlbSafe1_VehModMngtGlbSafe1_read : Inport  Port=623
  Vin_Vin_read : Inport  Port=624
  BswLifeCycle_LifeCycle_read : Inport  Port=625
  function : TriggerPort  TriggerType=function-call  VariantControl=(inherit)  SampleTimeType=periodic  SampleTime=-1
  Ground_{1..131} (131 alike) : Ground
  PA_NvMBlockCarCfgDataInit : Constant  Value=NvMBlockCarCfgDataInit
  PA_NvMBlockDidDataD005Init : Constant  Value=NvMBlockDidDataD005Init
  PA_NvMBlockDidDataD09AInit : Constant  Value=NvMBlockDidDataD09AInit
  PA_NvMBlockDidDataE103Init : Constant  Value=NvMBlockDidDataE103Init
  PA_NvMBlockDidDataF190Init : Constant  Value=NvMBlockDidDataF190Init
  PA_NvMBlockDidDataF1ABInit : Constant  Value=NvMBlockDidDataF1ABInit
  Terminator_{1..631} (631 alike) : Terminator
  CarCfgForClimaCtrl_CarCfgFor3RDROWSEATHEAT_write : Outport
      settings: EnsureOutportIsVirtual=on
  CarCfgForClimaCtrl_CarCfgFor3RDROWSEATVENTILATED_write : Outport  Port=2
      settings: EnsureOutportIsVirtual=on
  CarCfgForClimaCtrl_CarCfgForBRAND_write : Outport  Port=3
      settings: EnsureOutportIsVirtual=on
  CarCfgForClimaCtrl_CarCfgForCabinOxgenSupply_write : Outport  Port=4
      settings: EnsureOutportIsVirtual=on
  CarCfgForClimaCtrl_CarCfgForElectricLiftingModule_write : Outport  Port=5
      settings: EnsureOutportIsVirtual=on
  CarCfgForClimaCtrl_CarCfgForExtVentnClimaCtrl_write : Outport  Port=6
      settings: EnsureOutportIsVirtual=on
  CarCfgForClimaCtrl_CarCfgForTEMPEREDSTORAGEBOX_write : Outport  Port=7
      settings: EnsureOutportIsVirtual=on
  CarCfgForClimaCtrl_CarCfgForFloatingDriving_write : Outport  Port=8
      settings: EnsureOutportIsVirtual=on
  CarCfgForClimaCtrl_CarCfgForInteriorViewCamera_write : Outport  Port=9
      settings: EnsureOutportIsVirtual=on
  CarCfgForClimaCtrl_CarCfgForLifeDetection_write : Outport  Port=10
      settings: EnsureOutportIsVirtual=on
  CarCfgForClimaCtrl_CarCfgForMARKET_write : Outport  Port=11
      settings: EnsureOutportIsVirtual=on
  CarCfgForClimaCtrl_CarCfgForPowerSeats_write : Outport  Port=12
      settings: EnsureOutportIsVirtual=on
  CarCfgForClimaCtrl_CarCfgForPTCInFrontHVAC_write : Outport  Port=13
      settings: EnsureOutportIsVirtual=on
  CarCfgForClimaCtrl_CarCfgForRFKEY_write : Outport  Port=14
      settings: EnsureOutportIsVirtual=on
  CarCfgForClimaCtrl_CarCfgForVentLiftMotorExiSts_write : Outport  Port=15
      settings: EnsureOutportIsVirtual=on
  CarCfgForClimaCtrl_CarCfgForWadingAssistSystem_write : Outport  Port=16
      settings: EnsureOutportIsVirtual=on
  CarCfgForClimaCtrl_CarCfgWindFollowHumanMov_write : Outport  Port=17
      settings: EnsureOutportIsVirtual=on
  HvacCfgForClimaCtrl_HvacCfgForAQSTypes_write : Outport  Port=18
      settings: EnsureOutportIsVirtual=on
  HvacCfgForClimaCtrl_HvacCfgForHvacLowSideRETXVESts_write : Outport  Port=19
      settings: EnsureOutportIsVirtual=on
  HvacCfgForClimaCtrl_HvacCfgForHvacReBlowerType_write : Outport  Port=20
      settings: EnsureOutportIsVirtual=on
  HvacCfgForClimaCtrl_HvacCfgForHvacReVentPstn_write : Outport  Port=21
      settings: EnsureOutportIsVirtual=on
  HvacCfgForClimaCtrl_HvacCfgForHvacSnsrRCmptmtESts_write : Outport  Port=22
      settings: EnsureOutportIsVirtual=on
  HvacCfgForClimaCtrl_HvacCfgForHvacSnsrReHexESts_write : Outport  Port=23
      settings: EnsureOutportIsVirtual=on
  HvacCfgReqVersionReplace_HvacCfgReqVersionReplace_write : Outport  Port=24
      settings: EnsureOutportIsVirtual=on
  SysServCarCfgSts_SysServCCPLin2AQSSts_write : Outport  Port=25
      settings: EnsureOutportIsVirtual=on
  SysServCarCfgSts_SysServDUBlowerPwmSignTypeSts_write : Outport  Port=26
      settings: EnsureOutportIsVirtual=on
  SysServCarCfgSts_SysServDULin4HFAFStsH_write : Outport  Port=27
      settings: EnsureOutportIsVirtual=on
  SysServCarCfgSts_SysServDUReBlowerPwmSignSts_write : Outport  Port=28
      settings: EnsureOutportIsVirtual=on
  SysServCarCfgSts_SysServDUReBlowerPwmSts_write : Outport  Port=29
      settings: EnsureOutportIsVirtual=on
  SysServCarCfgSts_SysServFloatingMotorSts_write : Outport  Port=30
      settings: EnsureOutportIsVirtual=on
  SysServCarCfgSts_SysServFrntHEXSnsrPosn_write : Outport  Port=31
      settings: EnsureOutportIsVirtual=on
  SysServCarCfgSts_SysServLowSideRETXVSts_write : Outport  Port=32
      settings: EnsureOutportIsVirtual=on
  SysServCarCfgSts_SysServReHEXSnsrPosn_write : Outport  Port=33
      settings: EnsureOutportIsVirtual=on
  SysServCarCfgSts_SysServRHFASts_write : Outport  Port=34
      settings: EnsureOutportIsVirtual=on
  SysServCarCfgSts_SysServSnsrForFCmptmtTType_write : Outport  Port=35
      settings: EnsureOutportIsVirtual=on
  CarCfgForClimaCtrl_CarCfgForAutDemst_write : Outport  Port=36
      settings: EnsureOutportIsVirtual=on
  CarCfgForClimaCtrl_CarCfgForAirClng_write : Outport  Port=37
      settings: EnsureOutportIsVirtual=on
  CarCfgForClimaCtrl_CarCfgForAirClnrAdv_write : Outport  Port=38
      settings: EnsureOutportIsVirtual=on
  CarCfgForClimaCtrl_CarCfgForAirFrag_write : Outport  Port=39
      settings: EnsureOutportIsVirtual=on
  CarCfgForClimaCtrl_CarCfgForAirMtrlSnsr_write : Outport  Port=40
      settings: EnsureOutportIsVirtual=on
  CarCfgForClimaCtrl_CarCfgForAirQly_write : Outport  Port=41
      settings: EnsureOutportIsVirtual=on
  CarCfgForClimaCtrl_CarCfgForAmntOfSeat_write : Outport  Port=42
      settings: EnsureOutportIsVirtual=on
  CarCfgForClimaCtrl_CarCfgForAuxWtrPmp_write : Outport  Port=43
      settings: EnsureOutportIsVirtual=on
  CarCfgForClimaCtrl_CarCfgForBypVlvInHvac_write : Outport  Port=44
      settings: EnsureOutportIsVirtual=on
  CarCfgForClimaCtrl_CarCfgForClsVlvInHvac_write : Outport  Port=45
      settings: EnsureOutportIsVirtual=on
  CarCfgForClimaCtrl_CarCfgForCmprAcTyp_write : Outport  Port=46
      settings: EnsureOutportIsVirtual=on
  CarCfgForClimaCtrl_CarCfgForCmptmtClng_write : Outport  Port=47
      settings: EnsureOutportIsVirtual=on
  CarCfgForClimaCtrl_CarCfgForElecVentAdjmt_write : Outport  Port=48
      settings: EnsureOutportIsVirtual=on
  CarCfgForClimaCtrl_CarCfgForFlcTyp_write : Outport  Port=49
      settings: EnsureOutportIsVirtual=on
  CarCfgForClimaCtrl_CarCfgForFoldCSD_write : Outport  Port=50
      settings: EnsureOutportIsVirtual=on
  CarCfgForClimaCtrl_CarCfgForFuTyp_write : Outport  Port=51
      settings: EnsureOutportIsVirtual=on
  CarCfgForClimaCtrl_CarCfgForGlassTyp_write : Outport  Port=52
      settings: EnsureOutportIsVirtual=on
  CarCfgForClimaCtrl_CarCfgForHeadRadar_write : Outport  Port=53
      settings: EnsureOutportIsVirtual=on
  CarCfgForClimaCtrl_CarCfgForHeatPmp_write : Outport  Port=54
      settings: EnsureOutportIsVirtual=on
  CarCfgForClimaCtrl_CarCfgForHeatrDurgDrvg_write : Outport  Port=55
      settings: EnsureOutportIsVirtual=on
  CarCfgForClimaCtrl_CarCfgForHeatrTyp_write : Outport  Port=56
      settings: EnsureOutportIsVirtual=on
  CarCfgForClimaCtrl_CarCfgForHvacFilTyp_write : Outport  Port=57
      settings: EnsureOutportIsVirtual=on
  CarCfgForClimaCtrl_CarCfgForHvacFlrSnsrForT_write : Outport  Port=58
      settings: EnsureOutportIsVirtual=on
  CarCfgForClimaCtrl_CarCfgForHvacHeatrSnsrT_write : Outport  Port=59
      settings: EnsureOutportIsVirtual=on
  CarCfgForClimaCtrl_CarCfgForHvacRe_write : Outport  Port=60
      settings: EnsureOutportIsVirtual=on
  CarCfgForClimaCtrl_CarCfgForHvacTyp_write : Outport  Port=61
      settings: EnsureOutportIsVirtual=on
  CarCfgForClimaCtrl_CarCfgForLoVoltElecCfg_write : Outport  Port=62
      settings: EnsureOutportIsVirtual=on
  CarCfgForClimaCtrl_CarCfgForNavSysandIntnmap_write : Outport  Port=63
      settings: EnsureOutportIsVirtual=on
  CarCfgForClimaCtrl_CarCfgForNrOfDoor_write : Outport  Port=64
      settings: EnsureOutportIsVirtual=on
  CarCfgForClimaCtrl_CarCfgForPipeInFuTank_write : Outport  Port=65
      settings: EnsureOutportIsVirtual=on
  CarCfgForClimaCtrl_CarCfgForPreClng_write : Outport  Port=66
      settings: EnsureOutportIsVirtual=on
  CarCfgForClimaCtrl_CarCfgForPrkgCDSNotAvl_write : Outport  Port=67
      settings: EnsureOutportIsVirtual=on
  CarCfgForClimaCtrl_CarCfgForPrkgClima_write : Outport  Port=68
      settings: EnsureOutportIsVirtual=on
  CarCfgForClimaCtrl_CarCfgForPrpsnTyp_write : Outport  Port=69
      settings: EnsureOutportIsVirtual=on
  CarCfgForClimaCtrl_CarCfgForRemEngStrtPreCdn_write : Outport  Port=70
      settings: EnsureOutportIsVirtual=on
  CarCfgForClimaCtrl_CarCfgForRoofHatch_write : Outport  Port=71
      settings: EnsureOutportIsVirtual=on
  CarCfgForClimaCtrl_CarCfgForSeatHeatgCal_write : Outport  Port=72
      settings: EnsureOutportIsVirtual=on
  CarCfgForClimaCtrl_CarCfgForSeatHeatgForRowFirst_write : Outport  Port=73
      settings: EnsureOutportIsVirtual=on
  CarCfgForClimaCtrl_CarCfgForSeatHeatgForRowSec_write : Outport  Port=74
      settings: EnsureOutportIsVirtual=on
  CarCfgForClimaCtrl_CarCfgForSeatVentnForRowFirst_write : Outport  Port=75
      settings: EnsureOutportIsVirtual=on
  CarCfgForClimaCtrl_CarCfgForSeatVentnForRowSec_write : Outport  Port=76
      settings: EnsureOutportIsVirtual=on
  CarCfgForClimaCtrl_CarCfgForSecRowVentnCenter_write : Outport  Port=77
      settings: EnsureOutportIsVirtual=on
  CarCfgForClimaCtrl_CarCfgForSecRowVentnSide_write : Outport  Port=78
      settings: EnsureOutportIsVirtual=on
  CarCfgForClimaCtrl_CarCfgForSmallFlowCyc_write : Outport  Port=79
      settings: EnsureOutportIsVirtual=on
  CarCfgForClimaCtrl_CarCfgForSmkSnsr_write : Outport  Port=80
      settings: EnsureOutportIsVirtual=on
  CarCfgForClimaCtrl_CarCfgForSnsrForCmptmtT_write : Outport  Port=81
      settings: EnsureOutportIsVirtual=on
  CarCfgForClimaCtrl_CarCfgForSnsrHumPrsnt_write : Outport  Port=82
      settings: EnsureOutportIsVirtual=on
  CarCfgForClimaCtrl_CarCfgForStartDevice_write : Outport  Port=83
      settings: EnsureOutportIsVirtual=on
  CarCfgForClimaCtrl_CarCfgForSteerWhlHeatg_write : Outport  Port=84
      settings: EnsureOutportIsVirtual=on
  CarCfgForClimaCtrl_CarCfgForSteerWhlPosn_write : Outport  Port=85
      settings: EnsureOutportIsVirtual=on
  CarCfgForClimaCtrl_CarCfgForSunSnsr_write : Outport  Port=86
      settings: EnsureOutportIsVirtual=on
  CarCfgForClimaCtrl_CarCfgForTelmNodTyp_write : Outport  Port=87
      settings: EnsureOutportIsVirtual=on
  CarCfgForClimaCtrl_CarCfgForThermMngt_write : Outport  Port=88
      settings: EnsureOutportIsVirtual=on
  CarCfgForClimaCtrl_CarCfgForVehTyp_write : Outport  Port=89
      settings: EnsureOutportIsVirtual=on
  CarCfgForClimaCtrl_CarCfgForVgaMotorExistSts_write : Outport  Port=90
      settings: EnsureOutportIsVirtual=on
  CarCfgForClimaCtrl_CarCfgForWindHeatd_write : Outport  Port=91
      settings: EnsureOutportIsVirtual=on
  HvacCfgForClimaCtrl_HvacCfgForHvacFrontBlowerType_write : Outport  Port=92
      settings: EnsureOutportIsVirtual=on
  HvacCfgForClimaCtrl_HvacCfgForHvacMiscExistSts_write : Outport  Port=93
      settings: EnsureOutportIsVirtual=on
  HvacCfgForClimaCtrl_HvacCfgForHvacMotorExistSts_write : Outport  Port=94
      settings: EnsureOutportIsVirtual=on
  HvacCfgForClimaCtrl_HvacCfgForHvacSensorExistSts_write : Outport  Port=95
      settings: EnsureOutportIsVirtual=on
  SysServCarCfgSts_SysServCarID_write : Outport  Port=96
      settings: EnsureOutportIsVirtual=on
  SysServCarCfgSts_SysServCCPAQSSts_write : Outport  Port=97
      settings: EnsureOutportIsVirtual=on
  SysServCarCfgSts_SysServCCPBGMNum_write : Outport  Port=98
      settings: EnsureOutportIsVirtual=on
  SysServCarCfgSts_SysServCCPHEXSensorSts_write : Outport  Port=99
      settings: EnsureOutportIsVirtual=on
  SysServCarCfgSts_SysServCCPLin2AFUSts_write : Outport  Port=100
      settings: EnsureOutportIsVirtual=on
  SysServCarCfgSts_SysServCCPLin2AIUSts_write : Outport  Port=101
      settings: EnsureOutportIsVirtual=on
  SysServCarCfgSts_SysServCCPLin2CDSSts_write : Outport  Port=102
      settings: EnsureOutportIsVirtual=on
  SysServCarCfgSts_SysServCCPLin2PMSISts_write : Outport  Port=103
      settings: EnsureOutportIsVirtual=on
  SysServCarCfgSts_SysServCCPLin3VGASts_write : Outport  Port=104
      settings: EnsureOutportIsVirtual=on
  SysServCarCfgSts_SysServDUAirVentSnrSts_write : Outport  Port=105
      settings: EnsureOutportIsVirtual=on